simulink model "nodo_pos"  (R2020a)
settings: Solver=FixedStepDiscrete  StartTime=0.0  StopTime=inf  FixedStep=params.Ts  RelTol=1e-3  EnableMultiTasking=on
PreLoadFcn:
  | Init_pos;
model workspace (MAT data in the model file):
  SL_ROS_VariableLengthArrays_MaxSizes.CommonInfo.ArrayTruncateAction = 'EmitWarning'
  SL_ROS_VariableLengthArrays_MaxSizes.MsgTypeMap = containers.Map
  SL_ROS_VariableLengthArrays_MaxSizes.MsgTypeMap.serialization.keys = {'sensor_msgs/Image', 'sensor_msgs/LaserScan'}
  SL_ROS_VariableLengthArrays_MaxSizes.MsgTypeMap.serialization.values{1}(1).PropertyName = 'Data'
  SL_ROS_VariableLengthArrays_MaxSizes.MsgTypeMap.serialization.values{1}(1).DataType = 'uint8'
  SL_ROS_VariableLengthArrays_MaxSizes.MsgTypeMap.serialization.values{1}(1).MaxLength = 921600
  SL_ROS_VariableLengthArrays_MaxSizes.MsgTypeMap.serialization.values{1}(2).PropertyName = 'Encoding'
  SL_ROS_VariableLengthArrays_MaxSizes.MsgTypeMap.serialization.values{1}(2).DataType = 'uint8'
  SL_ROS_VariableLengthArrays_MaxSizes.MsgTypeMap.serialization.values{1}(2).MaxLength = 128
  SL_ROS_VariableLengthArrays_MaxSizes.MsgTypeMap.serialization.values{2}(1).PropertyName = 'Intensities'
  SL_ROS_VariableLengthArrays_MaxSizes.MsgTypeMap.serialization.values{2}(1).DataType = 'single'
  SL_ROS_VariableLengthArrays_MaxSizes.MsgTypeMap.serialization.values{2}(1).MaxLength = 360
  SL_ROS_VariableLengthArrays_MaxSizes.MsgTypeMap.serialization.values{2}(2).PropertyName = 'Ranges'
  SL_ROS_VariableLengthArrays_MaxSizes.MsgTypeMap.serialization.values{2}(2).DataType = 'single'
  SL_ROS_VariableLengthArrays_MaxSizes.MsgTypeMap.serialization.values{2}(2).MaxLength = 360
  SL_ROS_VariableLengthArrays_MaxSizes.MsgTypeMap.serialization.uniformity = 0
  SL_ROS_VariableLengthArrays_MaxSizes.MsgTypeMap.serialization.keyType = 'char'
  SL_ROS_VariableLengthArrays_MaxSizes.MsgTypeMap.serialization.valueType = 'any'
  net : net (value not decoded)
undefined symbols (not defined in the file or in its folder): Angular, Linear
libraries: robotlib x4, simulink x1
settings Model: Creator=scastro  LastModifiedBy=GoniR
settings SolverCC: PositivePriorityOrder=on
settings DataIOCC: ReturnWorkspaceOutputs=off
settings OptimizationCC: PassReuseOutputArgsAs=Individual arguments  MaxStackSize=64  EfficientTunableParamExpr=on
settings DebuggingCC: ParameterTunabilityLossMsg=error  ArithmeticOperatorsInVariantConditions=warning
settings HardwareCC: ProdBitPerLong=64  ProdHWDeviceType=Intel->x86-64 (Linux 64)
settings SFSimCC: SimUseLocalCustomCode=off  CustomCodeUndefinedFunction=UseInterfaceOnly
settings RTWCC: SystemTargetFile=ert.tlc  HardwareBoard=Robot Operating System (ROS)  TemplateMakefile=ert_default_tmf  GenerateReport=on  RTWUseLocalCustomCode=off  Toolchain=Catkin  IncludeHyperlinkInReport=on  LaunchReport=on  TargetLang=C++  GenerateTraceInfo=on  GenerateTraceReport=on  GenerateTraceReportSl=on  GenerateTraceReportSf=on  GenerateTraceReportEml=on
settings CodeAppCC: ForceParamTrailComments=on  IgnoreCustomStorageClasses=off  OperatorAnnotations=on  SimulinkDataObjDesc=on  SFDataObjDesc=on  MATLABFcnDesc=on  InsertBlockDesc=on  StateflowObjectComments=on  MATLABSourceComments=on
settings ERTTargetCC: SupportVariableSizeSignals=on

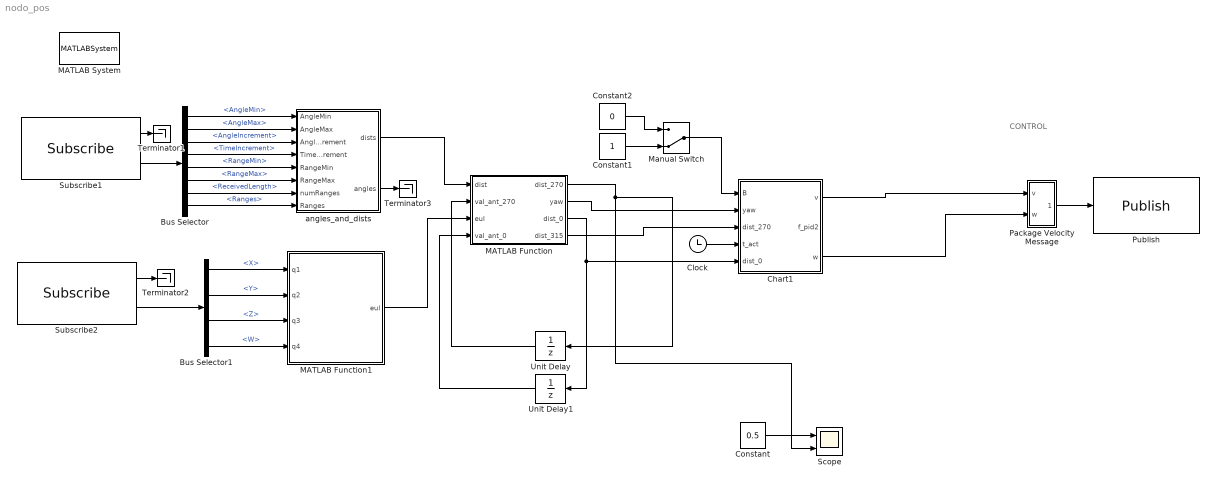
== system nodo_pos ==
  note: CONTROL
  Bus Selector : BusSelector  OutputSignals=AngleMin,AngleMax,AngleIncrement,TimeIncrement,RangeMin,RangeMax,Ranges_SL_Info.ReceivedLength,Ranges
  Bus Selector1 : BusSelector  OutputSignals=Pose.Pose.Orientation.X,Pose.Pose.Orientation.Y,Pose.Pose.Orientation.Z,Pose.Pose.Orientation.W
  Chart1 : Stateflow chart  TreatAsAtomicUnit=on
      settings: PortSchema="{\"entries\":[{\"content\":{\"sides\":[{\"content\":{\"connectorIds\":[\"In1\",\"In2\",\"In3\",\"In4\",\"In5\"]},\"type\":\"ConnectorPlacement.EquallySpacedRectSide\",\"uuid\":\"2f54c4e5-e0f1-4f55-9704-d0ca62598209\"},{\"content\":{\"connectorIds\":[\"Out2\",\"Out3\",\"Out1\"],\"side\":\"RIGHT\"},\"type\":\"ConnectorPlacement.EquallySpacedRectSide\",\"uuid\":\"05a61ef6-3f75-4aa5-9d08-afe380f82b00\"},{\"content\":{\"connectorIds\":[],\"side\":\"TOP\"},\"type\":\"ConnectorPlacement.EquallySpacedRectSide\",\"uuid\":\"70773613-5b33-4d60-86f5-82d79aa59450\"}]},\"type\":\"ConnectorPlacement.EquallySpacedConnector\",\"uuid\":\"58fa94d1-4180-4700-8989-c8987bd10f91\"}],\"packageUris\":[\"http://schema.mathworks.com/mf0/Connector/19700101\"],\"version\":\"1.0\"}"
  Clock : Clock
  Constant : Constant  Value=0.5
  Constant1 : Constant
  Constant2 : Constant  Value=0
  MATLAB Function : MATLAB Function  TreatAsAtomicUnit=on
  MATLAB Function1 : MATLAB Function  TreatAsAtomicUnit=on
  MATLAB System : MATLABSystem  System=ExampleHelperSimulationRateControl  SampleTime=0.02
      mask Type: ExampleHelperSimulationRateControl
      mask Display: disp('Rate Control');
  Manual Switch : ManualSwitch  CurrentSetting=0
  Package Velocity Message : Subsystem
  Publish : robotlib/Publish  topicSource=Select from ROS network  topic=/cmd_vel  messageType=geometry_msgs/Twist  messageQueueLength=1
  Scope : Scope  NumInputPorts=2
  Subscribe1 : robotlib/Subscribe  topicSource=Select from ROS network  topic=/scan  messageType=sensor_msgs/LaserScan  sampleTime=-1  messageQueueLength=1
  Subscribe2 : robotlib/Subscribe  topicSource=Select from ROS network  topic=/odom  messageType=nav_msgs/Odometry  sampleTime=-1  messageQueueLength=1
  Terminator1 : Terminator
  Terminator2 : Terminator
  Terminator3 : Terminator
  Unit Delay : UnitDelay  SampleTime=-1  HasFrameUpgradeWarning=on
  Unit Delay1 : UnitDelay  SampleTime=-1  HasFrameUpgradeWarning=on
  angles_and_dists : MATLAB Function  TreatAsAtomicUnit=on
  wires:
  Package Velocity Message/1(msg) -> Publish/1
  Subscribe1/1 -> Terminator1/1
  Bus Selector/8 -> angles_and_dists/8(Ranges)  "<Ranges>"
  Bus Selector/6 -> angles_and_dists/6(RangeMax)  "<RangeMax>"
  Bus Selector/2 -> angles_and_dists/2(AngleMax)  "<AngleMax>"
  Bus Selector/5 -> angles_and_dists/5(RangeMin)  "<RangeMin>"
  Bus Selector/3 -> angles_and_dists/3(AngleIncrement)  "<AngleIncrement>"
  Bus Selector/7 -> angles_and_dists/7(numRanges)  "<ReceivedLength>"
  Bus Selector/1 -> angles_and_dists/1(AngleMin)  "<AngleMin>"
  Bus Selector/4 -> angles_and_dists/4(TimeIncrement)  "<TimeIncrement>"
  Subscribe1/2 -> Bus Selector/1
  angles_and_dists/1(dists) -> MATLAB Function/1(dist)
  Chart1/2(v) -> Package Velocity Message/1(v)
  Chart1/1(w) -> Package Velocity Message/2(w)
  MATLAB Function/1(dist_270) -> Scope/2, Unit Delay/1
  Unit Delay/1 -> MATLAB Function/2(val_ant_270)
  Subscribe2/1 -> Terminator2/1
  Subscribe2/2 -> Bus Selector1/1
  Bus Selector1/1 -> MATLAB Function1/1(q1)  "<X>"
  Bus Selector1/2 -> MATLAB Function1/2(q2)  "<Y>"
  Bus Selector1/3 -> MATLAB Function1/3(q3)  "<Z>"
  Bus Selector1/4 -> MATLAB Function1/4(q4)  "<W>"
  MATLAB Function1/1(eul) -> MATLAB Function/3(eul)
  MATLAB Function/2(yaw) -> Chart1/2(yaw)
  angles_and_dists/2(angles) -> Terminator3/1
  Constant/1 -> Scope/1
  Manual Switch/1 -> Chart1/1(B)
  Constant1/1 -> Manual Switch/2
  Constant2/1 -> Manual Switch/1
  MATLAB Function/3(dist_0) -> Unit Delay1/1, Chart1/5(dist_0)
  Unit Delay1/1 -> MATLAB Function/4(val_ant_0)
  Clock/1 -> Chart1/4(t_act)
  MATLAB Function/4(dist_315) -> Chart1/3(dist_270)

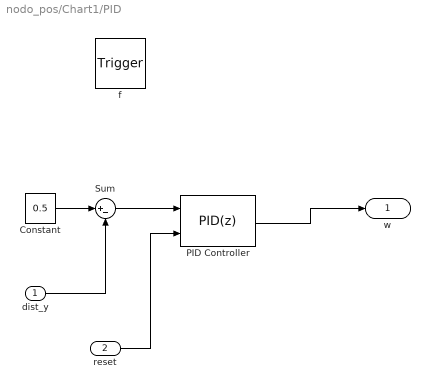
== system nodo_pos/Chart1/PID ==
  dist_y : Inport  OutDataTypeStr=double  PortDimensions=1
  reset : Inport  Port=2  OutDataTypeStr=double  PortDimensions=1
  f : TriggerPort  TriggerType=function-call  VariantControl=(inherit)
      settings: CopyFcn=Stateflow.SLINSF.SimulinkMan.removeBlockPreDeleteFcn  PreDeleteFcn=Stateflow.SLINSF.SimulinkMan.unsafePredeleteFcn
  Constant : Constant  Value=0.5
  PID Controller : simulink/Continuous/PID Controller  Controller=PID  Form=Parallel  TimeDomain=Discrete-time  UseExternalTs=off  SampleTime=-1  IntegratorMethod=Forward Euler  FilterMethod=Forward Euler  ControllerParametersSource=internal  P=0.5  I=0.01  D=0.09  UseFilter=on  N=100  TunerSelectOption=Transfer Function Based (PID Tuner App)  InitialConditionSource=internal  InitialConditionForIntegrator=0  InitialConditionForFilter=0  DifferentiatorICPrevScaledInput=0  ExternalReset=level  TrackingMode=off  Kt=1  LimitOutput=off  UpperSaturationLimit=inf  LowerSaturationLimit=-inf  LinearizeAsGain=on  AntiWindupMode=none  Kb=1  POutMin=[]  POutMax=[]  IOutMin=[]  IOutMax=[]  DOutMin=[]  DOutMax=[]  NOutMin=[]  NOutMax=[]  SumOutMin=[]  SumOutMax=[]  SaturationOutMin=[]  SaturationOutMax=[]  PParamMin=[]  PParamMax=[]  IParamMin=[]  IParamMax=[]  DParamMin=[]  DParamMax=[]  NParamMin=[]  NParamMax=[]  KbParamMin=[]  KbParamMax=[]  KtParamMin=[]  KtParamMax=[]  KbOutMin=[]  KbOutMax=[]  KtOutMin=[]  KtOutMax=[]  IntegratorOutMin=[]  IntegratorOutMax=[]  FilterOutMin=[]  FilterOutMax=[]  SumI1OutMin=[]  SumI1OutMax=[]  SumI2OutMin=[]  SumI2OutMax=[]  SumI3OutMin=[]  SumI3OutMax=[]  SumI4OutMin=[]  SumI4OutMax=[]  SumDOutMin=[]  SumDOutMax=[]  DifferentiatorOutMin=[]  DifferentiatorOutMax=[]  FilterDiffOutCoefMin=[]  FilterDiffOutCoefMax=[]  ReciprocalOutMin=[]  ReciprocalOutMax=[]  SumDenOutMin=[]  SumDenOutMax=[]  SumNumOutMin=[]  SumNumOutMax=[]  DivideOutMin=[]  DivideOutMax=[]  UdiffTsProdOutMin=[]  UdiffTsProdOutMax=[]  NTsProdOutMin=[]  NTsProdOutMax=[]  UintegralTsProdOutMin=[]  UintegralTsProdOutMax=[]  UngainTsProdOutMin=[]  UngainTsProdOutMax=[]  IntegratorContinuousStateAttributes=''  IntegratorStateMustResolveToSignalObject=off  IntegratorRTWStateStorageClass=Auto  FilterContinuousStateAttributes=''  FilterStateMustResolveToSignalObject=off  FilterRTWStateStorageClass=Auto  ParallelPVariant=InternalParameters  IdealPVariant=Disabled  IVariant=InternalParameters  DVariant=InternalParameters  IntegratorVariant=Discrete  SatVariant=Disabled  AWVariant=Passthrough  PCopyVariant=Disabled  TRVariant=Disabled  FdbkBlocksVariant=Forward  IdealPFdbkVariant=Disabled  SatFdbkVariant=Disabled  DerivativeFilterVariant=ForwardEulerFilter  NVariant=InternalParameters  NCopyVariant=Disabled  FilterICVariant=InternalICsFilter  IntegratorICVariant=InternalICs  ExternalResetVariant=ExternalReset  TRSumVariant=Passthrough  SumFdbkVariant=Disabled  SumVariant=Sum_PID  TsampFilterVariant=InternalTs  TsampNgainVariant=Passthrough  TsampIntegralVariant=Passthrough
  Sum : Sum  Inputs=|+-
  w : Outport
  wires:
  dist_y/1 -> Sum/2
  Constant/1 -> Sum/1
  Sum/1 -> PID Controller/1
  PID Controller/1 -> w/1
  reset/1 -> PID Controller/2

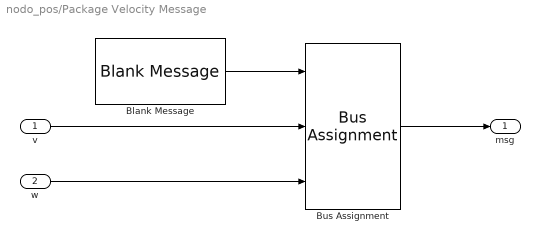
== system nodo_pos/Package Velocity Message ==
  v : Inport
  w : Inport  Port=2
  Blank Message : robotlib/Blank Message  messageClass=Message  entityType=geometry_msgs/Twist  messageType=geometry_msgs/Twist  SampleTime=inf
  Bus Assignment : BusAssignment  AssignedSignals=Linear.X,Angular.Z
  msg : Outport  VectorParamsAs1DForOutWhenUnconnected=off
  wires:
  Blank Message/1 -> Bus Assignment/1
  Bus Assignment/1 -> msg/1
  w/1 -> Bus Assignment/3
  v/1 -> Bus Assignment/2

== stateflow chart nodo_pos/Chart1 ==
  state Operativo
    state Marcha [parallel]
      settings: executionOrder=1
      state Seguir_pared
        en:
        f_pid=0;
        f_pid2=0;
        during:
        v=0.2;
        w=PID(dist_270,0);
      state Pared_delante
        state Girar
          en:
          temp_in=t_act;
          f_esperar=0;
          v=0;
          w=0.55;
          f_avan=0;
          du:
          if (t_act-temp_in)>=0.126
          w=0;
          f_avan=1;
          end
        state Esperar_girar
          en:
          temp_in=t_act;
          v=0;
          w=0;
          f_pid2=0;
          du:
          if (t_act-temp_in)>=0.042
          f_pid2=1;
          end
        state Esperar_avanzar
          en:
          w = PID(dist_270,1);
          temp_in=t_act;
          f_esperar=0;
          v=0;
          w=0;
          du:
          if (t_act-temp_in)>=0.042
          f_esperar=1;
          end
        Operativo.Marcha.Pared_delante.Esperar_avanzar -> Operativo.Marcha.Pared_delante.Girar : [f_esperar]
        Operativo.Marcha.Pared_delante.Girar -> Operativo.Marcha.Pared_delante.Esperar_girar : [f_avan]
        (default) -> Operativo.Marcha.Pared_delante.Esperar_avanzar
      state No_pared
        state Avanzar
          en:
          w = PID(dist_270,1);
          temp_in=t_act;
          f_esperar=0;
          v=0.2;
          w=0;
          f_girar=0;
          du:
          if (t_act-temp_in)>=0.084
          v=0;
          f_girar=1;
          end
        state Girar
          en:
          temp_in=t_act;
          f_esperar=0;
          v=0;
          w=-0.55;
          f_avan=0;
          du:
          if (t_act-temp_in)>=0.126
          w=0;
          f_avan=1;
          end
        state Avanzar2
          en:
          temp_in=t_act;
          v=0.2;
          w=0;
          f_pid=0;
          du:
          if (t_act-temp_in)>=0.084
          v=0;
          f_pid=1;
          end
        state Esperar_avanzar
          en:
          temp_in=t_act;
          v=0;
          w=0;
          f_esperar=0;
          du:
          if (t_act-temp_in)>=0.042
          f_esperar=1;
          end
        state Esperar_girar
          en:
          temp_in=t_act;
          v=0;
          w=0;
          f_esperar=0;
          du:
          if (t_act-temp_in)>=0.042
          f_esperar=1;
          end
        (default) -> Avanzar
        Operativo.Marcha.No_pared.Esperar_avanzar -> Operativo.Marcha.No_pared.Girar : [f_esperar]
        Operativo.Marcha.No_pared.Esperar_girar -> Avanzar2 : [f_esperar]
        Operativo.Marcha.No_pared.Girar -> Operativo.Marcha.No_pared.Esperar_girar : [f_avan]
        Avanzar -> Operativo.Marcha.No_pared.Esperar_avanzar : [f_girar]
      (default) -> Seguir_pared
      Seguir_pared -> Pared_delante [order 2] : [dist_0<0.5 && dist_0>0]
      Pared_delante -> Seguir_pared : [f_pid2]
      Seguir_pared -> No_pared [order 1] : [dist_270==-1 || dist_270>0.8]
      No_pared -> Seguir_pared : [f_pid]
  state Detenido
    en:
    w = PID(dist_270,1);
    w=0;
    during:
    v=0;
    w=0;
  state w = PID(dist_y,reset) [function]
  (default) -> Detenido
  Detenido -> Operativo : [B]
  Operativo -> Detenido : [~B]
  data: w function_output; dist_y function_input double size=1; reset function_input double size=1; w output; v output; B input; yaw input; e local; cont local; flag local; dist_270 input; t_act input; temp_in local; f_avan local; f_girar local; f_pid local; f_esperar local; dist_0 input; f_pid2 output

== matlab function nodo_pos/angles_and_dists ==
  data: AngleMin input size=1 [-2*pi..2*pi]; dists output size=[360 1]; AngleMax input [-2*pi..2*pi]; AngleIncrement input [0..2*pi]; TimeIncrement input; RangeMin input; RangeMax input; numRanges input [1..360]; Ranges input; angles output size=[360 1]
function [dists, angles] = fcn(AngleMin, AngleMax, AngleIncrement, ...
                TimeIncrement, RangeMin, RangeMax, numRanges, Ranges)
%#codegen
% this function transforms the laser scan into cartesian coordinates, so
% that you can use other tools to group them together, make an image, etc.
if numRanges > 0 
angleMin = double(AngleMin);
angleMax = double(AngleMax);
angleIncrement = double(AngleIncrement);
% assert(angleMax > angleMin);
% assert((angleMax-angleMin)/angleIncrement > 1);
%angleMin = -pi/2;
%angleMax = pi/2;
%angleIncrement = pi/179;
%timeIncrement = TimeIncrement;
%rangeMin = RangeMin;
%rangeMax = RangeMax;
ranges = Ranges;
ranges(ranges>RangeMax) = NaN;
ranges(ranges<RangeMin) = NaN;
%rangesLength = scan.Ranges_SL_Info.ReceivedLength;
%intensities = scan.Intensities;
%coder.varsize('angles',[180 1]);
assert(numRanges <= 360);
angles=double(zeros(numRanges,1));
for ii=1:numRanges
    angles(ii) = double(angleMin) + double(ii-1)*(angleIncrement);
end
% angles = [angleMin:angleIncrement:angleMax]';
dists = ranges;
assert(length(dists) == numRanges);
assert(length(dists) == length(angles));
else
    angles=double(0);
    dists=single(0);
end

== matlab function nodo_pos/MATLAB Function ==
  data: dist input; dist_270 output; val_ant_270 input; eul input; yaw output; val_ant_0 input; dist_0 output; dist_315 output
function [dist_270,yaw,dist_0,dist_315]=detect_slam(dist,val_ant_270,eul,val_ant_0)
dist=double(dist);
%Compensamos con angulos de euler
yaw = round(rad2deg(eul(3)));
%Detección de distancia a pared frontal
%Detección de distancia a pared lateral y valor mínimo
dist_270=dist(270);
dist_315=dist(300);
dist_0=dist(1);
%Saturación
if dist_270>3.5
    dist_270=3.5;
end
if isnan(dist_270)
    dist_270=-1;
end
if dist_0>3.5
    dist_0=3.5;
end
if isnan(dist_0)
    dist_0=-1;
end
if dist_315>3.5
    dist_315=3.5;
end
if isnan(dist_315)
    dist_315=-1;
end

== matlab function nodo_pos/MATLAB Function1 ==
  data: q1 input; eul output; q2 input; q3 input; q4 input
function eul = fcn(q1,q2,q3,q4)
q = double([q1 q2 q3 q4]);
eul=quat2eul(q);
